simulink model "x64_32s_001"  (Simulink 7.4)
settings: Solver=ode45  StartTime=0.0  StopTime=100000  RelTol=1e-3
undefined symbols (not defined in the file): gcbh, ROACH, x64_32s_001_ct_sel_reg_user_data_out, x64_32s_001_inst_seng_id0_user_data_in, x64_32s_001_qdr_in_cnt_user_data_in, x64_32s_001_status_user_data_in, x64_32s_001_xaui_cnt0_user_data_in, x64_32s_001_xaui_error_cnt0_user_data_in, x64_32s_001_xauibuf_out_cnt_user_data_in, x64_32s_001_xauibuf_window_cnt_user_data_in, xlBlockLoadCallback
libraries: xbsIndex_r4 x7380, casper_library_misc x261, casper_library_delays x16, xps_library x6, ox_lib x1
(part 9 of 91)

== system x64_32s_001/vacc_monitor1 ==
  cnt_rst : Inport
  error0 : Inport  Port=2
  Delay25 : Subsystem  mask(pipeline){latency=1}
      mask help:
      | An explicitly laid-out delay line to avoid mapping to SRL16s
      | for use in pipelining to help achieve timing closure.
      mask init:
      | pipeline_init(gcb, 'latency', latency);
      mask Display:
      | port_label('input', 1, 'd');
      | port_label('output', 1, 'q');
      | disp(['Z^-', num2str(latency)]);
  payload_cnt1 : S-Function  LoadFcn=xlBlockLoadCallback(gcbh);  FunctionName=sysgen  Parameters=ptable_  EnableBusSupport=off  mask(Xilinx Counter Block){infoedit=Hardware notes: Free running counters are the least expensive in hardware.  A count limited counter is implemented by combining a counter with a comparator., cnt_type_sgadvanced=, cnt_type=Free Running, cnt_to=0, operation_sgadvanced=, operation=Up, start_count=0, cnt_by_val=1, arith_type_sgadvanced=, arith_type=Unsigned, n_bits=32, bin_pt=0, load_pin_sgadvanced=, load_pin=off, rst_sgadvanced=, rst=on, en_sgadvanced=, en=on, explicit_period_sgadvanced=, explicit_period=off, period=1, dbl_ovrd_sgadvanced=, dbl_ovrd=off, use_behavioral_HDL_sgadvanced=, use_behavioral_HDL=off, implementation_sgadvanced=, implementation=Fabric, xl_use_area_sgadvanced=, xl_use_area=off, xl_area=[17 32 0 32 0 0 0], use_rpm=on, xlcconfig=, has_advanced_control=0, sggui_pos=20,20,348,618, block_type=counter, block_version=10.1.3, sg_icon_stat=30,35,2,1,white,blue,0,d605779c,right,, sg_mask_display=|, sg_list_contents=, sg_blockgui_xml=}
      mask sg_mask_display=|
      | fprintf('','COMMENT: begin icon graphics');
      | patch([0 1 1 0 ],[0 0 1 1 ],[0.77 0.82 0.91]);
      | patch([0.233333 0.0666667 0.3 0.0666667 0.233333 0.5 0.566667 0.633333 0.9 0.666667 0.466667 0.3 0.5 0.3 0.466667 0.666667 0.9 0.633333 0.566667 0.5 0.233333 ],[0.171429 0.314286 0.514286 0.714286 0.857143 0.857143 0.8 0.857143 0.857143 0.657143 0.828571 0.685714 0.514286 0.342857 0.2 0.371429 0.171429 0.171429 0.228571 0.171429 0.171429 ],[0.98 0.96 0.92]);
      | plot([0 1 1 0 0 ],[0 0 1 1 0 ]);
      | fprintf('','COMMENT: end icon graphics');
      | fprintf('','COMMENT: begin icon text');
      | color('black');port_label('input',1,'rst');
      | color('black');port_label('input',2,'en');
      | color('black');port_label('output',1,'out');
      | fprintf('','COMMENT: end icon text');
      mask prompts: infoedit ""; cnt_type_sgadvanced ""; cnt_type "Counter type"; cnt_to "Count to value"; operation_sgadvanced ""; operation "Count direction"; start_count "Initial value"; cnt_by_val "Step"; arith_type_sgadvanced ""; arith_type "Output type"; n_bits "Number of bits"; bin_pt "Binary point"; load_pin_sgadvanced ""; load_pin "Provide load port"; rst_sgadvanced ""; rst "Provide synchronous reset port"; en_sgadvanced ""; en "Provide enable port"; explicit_period_sgadvanced ""; explicit_period "Sample period source"; period "Explicit period"; dbl_ovrd_sgadvanced ""; dbl_ovrd "Override with doubles"; use_behavioral_HDL_sgadvanced ""; use_behavioral_HDL "Use behavioral HDL (otherwise use core)"; implementation_sgadvanced ""; implementation "Implement using"; xl_use_area_sgadvanced ""; xl_use_area "Define FPGA area for resource estimation"; xl_area "FPGA area [slices, FFs, BRAMs, LUTs, IOBs, emb. mults, TBUFs]"; use_rpm ""; xlcconfig ""; has_advanced_control ""; sggui_pos ""; block_type ""; block_version ""; sg_icon_stat ""; sg_mask_display ""; sg_list_contents ""; sg_blockgui_xml ""
      mask help: Hardware notes: Free running counters are the least expensive in hardware. A count limited counter is implemented by combining a counter with a comparator.
      mask init: same as x64_32s_001/payload_cnt1
      settings: CopyFcn=xlBlockMoveCallback(gcbh);  DeleteFcn=xlDestroyGui(gcbh);  ModelCloseFcn=xlDestroyGui(gcbh);  PreSaveFcn=xlBlockPreSaveCallback(gcbh);  PostSaveFcn=xlBlockPostSaveCallback(gcbh);  DestroyFcn=xlDestroyGui(gcbh);  OpenFcn=xlOpenGui(gcbh, 'counter_gui.xml', @counterenablement, -1)  CloseFcn=xlDestroyGui(gcbh);  MoveFcn=xlBlockMoveCallback(gcbh);
      mask Help: eval('');xlDoc('-book','sysgen','-topic','Counter');
      mask StyleString: edit,edit,popup(Free Running|Count Limited),edit,edit,popup(Up|Down|Up/Down),edit,edit,edit,popup(Signed  (2's comp)|Unsigned),edit,edit,edit,checkbox,edit,checkbox,edit,checkbox,edit,popup(on|off),edit,edit,checkbox,edit,checkbox,edit,popup(Fabric|DSP48),edit,checkbox,edit,edit,edit,edit,edit,edit,edit,edit,edit,edit,edit
      mask Display:
      | fprintf('','COMMENT: begin icon graphics');
      | patch([0 1 1 0 ],[0 0 1 1 ],[0.77 0.82 0.91]);
      | patch([0.233333 0.0666667 0.3 0.0666667 0.233333 0.5 0.566667 0.633333 0.9 0.666667 0.466667 0.3 0.5 0.3 0.466667 0.666667 0.9 0.633333 0.566667 0.5 0.233333 ],[0.171429 0.314286 0.514286 0.714286 0.857143 0.857143 0.8 0.857143 0.857143 0.657143 0.828571 0.685714 0.514286 0.342857 0.2 0.371429 0.171429 0.171429 0.228571 0.171429 0.171429 ],[0.98 0.96 0.92]);
      | plot([0 1 1 0 0 ],[0 0 1 1 0 ]);
      | fprintf('','COMMENT: end icon graphics');
      | fprintf('','COMMENT: begin icon text');
      | color('black');port_label('input',1,'rst');
      | color('black');port_label('input',2,'en');
      | color('black');port_label('output',1,'out');
      | fprintf('','COMMENT: end icon text');
  cnt0 : Outport
  wires:
  error0/1 -> payload_cnt1/2
  Delay25/1(q) -> cnt0/1
  cnt_rst/1 -> payload_cnt1/1
  payload_cnt1/1 -> Delay25/1(d)

== system x64_32s_001/vacc_monitor2 ==
  cnt_rst : Inport
  error0 : Inport  Port=2
  Delay25 : Subsystem  mask(pipeline){latency=1}
      mask help:
      | An explicitly laid-out delay line to avoid mapping to SRL16s
      | for use in pipelining to help achieve timing closure.
      mask init:
      | pipeline_init(gcb, 'latency', latency);
      mask Display:
      | port_label('input', 1, 'd');
      | port_label('output', 1, 'q');
      | disp(['Z^-', num2str(latency)]);
  payload_cnt1 : S-Function  LoadFcn=xlBlockLoadCallback(gcbh);  FunctionName=sysgen  Parameters=ptable_  EnableBusSupport=off  mask(Xilinx Counter Block){infoedit=Hardware notes: Free running counters are the least expensive in hardware.  A count limited counter is implemented by combining a counter with a comparator., cnt_type_sgadvanced=, cnt_type=Free Running, cnt_to=0, operation_sgadvanced=, operation=Up, start_count=0, cnt_by_val=1, arith_type_sgadvanced=, arith_type=Unsigned, n_bits=32, bin_pt=0, load_pin_sgadvanced=, load_pin=off, rst_sgadvanced=, rst=on, en_sgadvanced=, en=on, explicit_period_sgadvanced=, explicit_period=off, period=1, dbl_ovrd_sgadvanced=, dbl_ovrd=off, use_behavioral_HDL_sgadvanced=, use_behavioral_HDL=off, implementation_sgadvanced=, implementation=Fabric, xl_use_area_sgadvanced=, xl_use_area=off, xl_area=[17 32 0 32 0 0 0], use_rpm=on, xlcconfig=, has_advanced_control=0, sggui_pos=20,20,348,618, block_type=counter, block_version=10.1.3, sg_icon_stat=30,35,2,1,white,blue,0,d605779c,right,, sg_mask_display=|, sg_list_contents=, sg_blockgui_xml=}
      mask sg_mask_display=|
      | fprintf('','COMMENT: begin icon graphics');
      | patch([0 1 1 0 ],[0 0 1 1 ],[0.77 0.82 0.91]);
      | patch([0.233333 0.0666667 0.3 0.0666667 0.233333 0.5 0.566667 0.633333 0.9 0.666667 0.466667 0.3 0.5 0.3 0.466667 0.666667 0.9 0.633333 0.566667 0.5 0.233333 ],[0.171429 0.314286 0.514286 0.714286 0.857143 0.857143 0.8 0.857143 0.857143 0.657143 0.828571 0.685714 0.514286 0.342857 0.2 0.371429 0.171429 0.171429 0.228571 0.171429 0.171429 ],[0.98 0.96 0.92]);
      | plot([0 1 1 0 0 ],[0 0 1 1 0 ]);
      | fprintf('','COMMENT: end icon graphics');
      | fprintf('','COMMENT: begin icon text');
      | color('black');port_label('input',1,'rst');
      | color('black');port_label('input',2,'en');
      | color('black');port_label('output',1,'out');
      | fprintf('','COMMENT: end icon text');
      mask prompts: infoedit ""; cnt_type_sgadvanced ""; cnt_type "Counter type"; cnt_to "Count to value"; operation_sgadvanced ""; operation "Count direction"; start_count "Initial value"; cnt_by_val "Step"; arith_type_sgadvanced ""; arith_type "Output type"; n_bits "Number of bits"; bin_pt "Binary point"; load_pin_sgadvanced ""; load_pin "Provide load port"; rst_sgadvanced ""; rst "Provide synchronous reset port"; en_sgadvanced ""; en "Provide enable port"; explicit_period_sgadvanced ""; explicit_period "Sample period source"; period "Explicit period"; dbl_ovrd_sgadvanced ""; dbl_ovrd "Override with doubles"; use_behavioral_HDL_sgadvanced ""; use_behavioral_HDL "Use behavioral HDL (otherwise use core)"; implementation_sgadvanced ""; implementation "Implement using"; xl_use_area_sgadvanced ""; xl_use_area "Define FPGA area for resource estimation"; xl_area "FPGA area [slices, FFs, BRAMs, LUTs, IOBs, emb. mults, TBUFs]"; use_rpm ""; xlcconfig ""; has_advanced_control ""; sggui_pos ""; block_type ""; block_version ""; sg_icon_stat ""; sg_mask_display ""; sg_list_contents ""; sg_blockgui_xml ""
      mask help: Hardware notes: Free running counters are the least expensive in hardware. A count limited counter is implemented by combining a counter with a comparator.
      mask init: same as x64_32s_001/payload_cnt1
      settings: CopyFcn=xlBlockMoveCallback(gcbh);  DeleteFcn=xlDestroyGui(gcbh);  ModelCloseFcn=xlDestroyGui(gcbh);  PreSaveFcn=xlBlockPreSaveCallback(gcbh);  PostSaveFcn=xlBlockPostSaveCallback(gcbh);  DestroyFcn=xlDestroyGui(gcbh);  OpenFcn=xlOpenGui(gcbh, 'counter_gui.xml', @counterenablement, -1)  CloseFcn=xlDestroyGui(gcbh);  MoveFcn=xlBlockMoveCallback(gcbh);
      mask Help: eval('');xlDoc('-book','sysgen','-topic','Counter');
      mask StyleString: edit,edit,popup(Free Running|Count Limited),edit,edit,popup(Up|Down|Up/Down),edit,edit,edit,popup(Signed  (2's comp)|Unsigned),edit,edit,edit,checkbox,edit,checkbox,edit,checkbox,edit,popup(on|off),edit,edit,checkbox,edit,checkbox,edit,popup(Fabric|DSP48),edit,checkbox,edit,edit,edit,edit,edit,edit,edit,edit,edit,edit,edit
      mask Display:
      | fprintf('','COMMENT: begin icon graphics');
      | patch([0 1 1 0 ],[0 0 1 1 ],[0.77 0.82 0.91]);
      | patch([0.233333 0.0666667 0.3 0.0666667 0.233333 0.5 0.566667 0.633333 0.9 0.666667 0.466667 0.3 0.5 0.3 0.466667 0.666667 0.9 0.633333 0.566667 0.5 0.233333 ],[0.171429 0.314286 0.514286 0.714286 0.857143 0.857143 0.8 0.857143 0.857143 0.657143 0.828571 0.685714 0.514286 0.342857 0.2 0.371429 0.171429 0.171429 0.228571 0.171429 0.171429 ],[0.98 0.96 0.92]);
      | plot([0 1 1 0 0 ],[0 0 1 1 0 ]);
      | fprintf('','COMMENT: end icon graphics');
      | fprintf('','COMMENT: begin icon text');
      | color('black');port_label('input',1,'rst');
      | color('black');port_label('input',2,'en');
      | color('black');port_label('output',1,'out');
      | fprintf('','COMMENT: end icon text');
  cnt0 : Outport
  wires:
  payload_cnt1/1 -> Delay25/1(d)
  cnt_rst/1 -> payload_cnt1/1
  Delay25/1(q) -> cnt0/1
  error0/1 -> payload_cnt1/2

== system x64_32s_001/vacc_sim_mux ==
  sync : Inport  OutDataType=fixdt(1, 16)  OutScaling=2^0
  vacc_din : Inport  Port=2  OutDataType=fixdt(1, 16)  OutScaling=2^0
  vld : Inport  Port=3  OutDataType=fixdt(1, 16)  OutScaling=2^0
  Counter : xbsIndex_r4/Counter  cnt_type=Free Running  cnt_to=Inf  operation=Up  start_count=0  cnt_by_val=1  arith_type=Unsigned  n_bits=3  bin_pt=0  load_pin=off  rst=on  en=off  explicit_period=on  period=1  dbl_ovrd=off  use_behavioral_HDL=off  implementation=Fabric  block_type=counter
      settings: block_version=11.4
  dout_sim : xbsIndex_r4/Simulation Multiplexer  sim_sel=1  hw_sel=2  block_type=simmux
      settings: infoedit="Distinguish input subsystems as \"simulation only\" and \"generation only\".  The input specified For Simulation will be used during Simulink simulation.  The input specified For Generation will be used during code generation.  This block will typically be used on the output of a HW Co-Simulation, Black Box, or ModelSim subsystem.<br><br>Hardware Notes: This block costs nothing."  block_version=11.4
  duplicate_16 : Subsystem
  pulse_ext : casper_library_misc/pulse_ext  SystemSampleTime=-1  pulse_len=8
  valid_sim : xbsIndex_r4/Simulation Multiplexer  sim_sel=1  hw_sel=2  block_type=simmux
      settings: infoedit="Distinguish input subsystems as \"simulation only\" and \"generation only\".  The input specified For Simulation will be used during Simulink simulation.  The input specified For Generation will be used during code generation.  This block will typically be used on the output of a HW Co-Simulation, Black Box, or ModelSim subsystem.<br><br>Hardware Notes: This block costs nothing."  block_version=11.4
  sync_out : Outport
  dout : Outport  Port=2
  vld_out : Outport  Port=3
  wires:
  vld/1 -> valid_sim/2
  valid_sim/1 -> vld_out/1
  sync/1 -> sync_out/1, Counter/1, pulse_ext/1
  dout_sim/1 -> dout/1
  vacc_din/1 -> dout_sim/2
  Counter/1 -> duplicate_16/1(In1)
  pulse_ext/1 -> valid_sim/1
  duplicate_16/1(Out1) -> dout_sim/1

== system x64_32s_001/x_window ==
  sync : Inport
  In1 : Inport  Port=2
  In2 : Inport  Port=3
  In3 : Inport  Port=4
  In4 : Inport  Port=5
  Delay : xbsIndex_r4/Delay  en=off  latency=5  dbl_ovrd=off  reg_retiming=on  block_type=delay
      settings: xl_area=[0, 0, 0, 0, 0, 0, 0]  block_version=9.2.01
  mult_0 : Subsystem  mask{coeff_width=18, input_width=4, output_width=18}
      mask prompts: coeff_width "Coefficient width?"; input_width "Input width?"; output_width "Output width?"
  mult_1 : Subsystem  mask{coeff_width=18, input_width=4, output_width=18}
      mask prompts: coeff_width "Coefficient width?"; input_width "Input width?"; output_width "Output width?"
  mult_2 : Subsystem  mask{coeff_width=18, input_width=4, output_width=18}
      mask prompts: coeff_width "Coefficient width?"; input_width "Input width?"; output_width "Output width?"
  mult_3 : Subsystem  mask{coeff_width=18, input_width=4, output_width=18}
      mask prompts: coeff_width "Coefficient width?"; input_width "Input width?"; output_width "Output width?"
  sync_out : Outport
  Out1 : Outport  Port=2
  Out2 : Outport  Port=3
  Out3 : Outport  Port=4
  Out4 : Outport  Port=5
  wires:
  In1/1 -> mult_0/1(in)
  mult_0/1(out) -> Out1/1
  In2/1 -> mult_1/1(in)
  mult_1/1(out) -> Out2/1
  In3/1 -> mult_2/1(in)
  mult_2/1(out) -> Out3/1
  In4/1 -> mult_3/1(in)
  mult_3/1(out) -> Out4/1
  sync/1 -> Delay/1
  Delay/1 -> sync_out/1

== system x64_32s_001/xaui_TVG ==
  din : Inport
  oob : Inport  Port=2
  empty : Inport  Port=3
  vld : Inport  Port=4
  ld : Inport  Port=5
  full : Inport  Port=6
  tvg_en : Inport  Port=7
  Constant1 : xbsIndex_r4/Constant  arith_type=Boolean  const=0  n_bits=36  bin_pt=0  explicit_period=on  period=1  equ=P=C  opselect=C  inp2=PCIN>>17  opr=+  inp1=P  carry=CIN  dbl_ovrd=off  block_type=constant
      settings: block_version=11.4
  Constant2 : xbsIndex_r4/Constant  arith_type=Boolean  const=0  n_bits=36  bin_pt=0  explicit_period=on  period=1  equ=P=C  opselect=C  inp2=PCIN>>17  opr=+  inp1=P  carry=CIN  dbl_ovrd=off  block_type=constant
      settings: block_version=11.4
  Constant4 : xbsIndex_r4/Constant  arith_type=Boolean  const=0  n_bits=36  bin_pt=0  explicit_period=on  period=1  equ=P=C  opselect=C  inp2=PCIN>>17  opr=+  inp1=P  carry=CIN  dbl_ovrd=off  block_type=constant
      settings: block_version=11.4
  dout-mux : xbsIndex_r4/Mux  inputs=2  en=off  latency=1  precision=Full  arith_type=Unsigned  n_bits=16  bin_pt=14  quantization=Truncate  overflow=Wrap  dbl_ovrd=off  block_type=mux
      settings: block_version=11.4
  edge_detect : casper_library_misc/edge_detect  SystemSampleTime=-1  edge=Rising  polarity=Active High  x_in=[0 0.08 0.08 0.16 0.16 0.24 0.24 0.32 0.32 0.4]  y_in="[-1 -1  1  1  1  1  1  1  1  1]"  x_out=[0.6 0.68 0.68 0.76 0.76 0.84 0.84 0.92 0.92 1]  y_out="[-1 -1  1  1 -1 -1 -1 -1 -1 -1]"
  empty_mux : xbsIndex_r4/Mux  inputs=2  en=off  latency=1  precision=Full  arith_type=Unsigned  n_bits=16  bin_pt=14  quantization=Truncate  overflow=Wrap  dbl_ovrd=off  block_type=mux
      settings: block_version=11.4
  full_mux : xbsIndex_r4/Mux  inputs=2  en=off  latency=1  precision=Full  arith_type=Unsigned  n_bits=16  bin_pt=14  quantization=Truncate  overflow=Wrap  dbl_ovrd=off  block_type=mux
      settings: block_version=11.4
  linkdown_mux : xbsIndex_r4/Mux  inputs=2  en=off  latency=1  precision=Full  arith_type=Unsigned  n_bits=16  bin_pt=14  quantization=Truncate  overflow=Wrap  dbl_ovrd=off  block_type=mux
      settings: block_version=11.4
  oob_mux : xbsIndex_r4/Mux  inputs=2  en=off  latency=1  precision=Full  arith_type=Unsigned  n_bits=16  bin_pt=14  quantization=Truncate  overflow=Wrap  dbl_ovrd=off  block_type=mux
      settings: block_version=11.4
  s_eng_TVG : Subsystem
  vld_mux : xbsIndex_r4/Mux  inputs=2  en=off  latency=1  precision=Full  arith_type=Unsigned  n_bits=16  bin_pt=14  quantization=Truncate  overflow=Wrap  dbl_ovrd=off  block_type=mux
      settings: block_version=11.4
  dout : Outport
  oob_out : Outport  Port=2
  empty_out : Outport  Port=3
  vld_out : Outport  Port=4
  ld_out : Outport  Port=5
  full_out : Outport  Port=6
  wires:
  edge_detect/1 -> s_eng_TVG/1(rst)
  s_eng_TVG/1(dout) -> dout-mux/3
  s_eng_TVG/2(vld) -> oob_mux/3
  s_eng_TVG/3(oob) -> vld_mux/3
  tvg_en/1 -> dout-mux/1, oob_mux/1, vld_mux/1, empty_mux/1, edge_detect/1, linkdown_mux/1, full_mux/1
  din/1 -> dout-mux/2
  dout-mux/1 -> dout/1
  empty/1 -> empty_mux/2
  empty_mux/1 -> empty_out/1
  linkdown_mux/1 -> ld_out/1
  full_mux/1 -> full_out/1
  ld/1 -> linkdown_mux/2
  full/1 -> full_mux/2
  Constant4/1 -> empty_mux/3
  Constant1/1 -> linkdown_mux/3
  Constant2/1 -> full_mux/3
  vld_mux/1 -> oob_out/1
  oob_mux/1 -> vld_out/1
  vld/1 -> oob_mux/2
  oob/1 -> vld_mux/2

== system x64_32s_001/xaui_buf0 ==
  din : Inport
  valid : Inport  Port=2
  hdr_valid : Inport  Port=3
  eof_in : Inport  Port=4
  hdr_in : Inport  Port=5
  Constant8 : xbsIndex_r4/Constant  arith_type=Boolean  const=0  n_bits=1  bin_pt=0  explicit_period=on  period=1  equ=P=C  opselect=C  inp2=PCIN>>17  opr=+  inp1=P  carry=CIN  dbl_ovrd=off  block_type=constant
  Delay39 : Subsystem  AncestorBlock=casper_library/Delays/pipeline  mask(pipeline){latency=1}
      mask help:
      | An explicitly laid-out delay line to avoid mapping to SRL16s
      | for use in pipelining to help achieve timing closure.
      mask init:
      | pipeline_init(gcb, 'latency', latency);
      mask Display:
      | port_label('input', 1, 'd');
      | port_label('output', 1, 'q');
      | disp(['Z^-', num2str(latency)]);
  Delay40 : Subsystem  AncestorBlock=casper_library/Delays/pipeline  mask(pipeline){latency=1}
      mask help:
      | An explicitly laid-out delay line to avoid mapping to SRL16s
      | for use in pipelining to help achieve timing closure.
      mask init:
      | pipeline_init(gcb, 'latency', latency);
      mask Display:
      | port_label('input', 1, 'd');
      | port_label('output', 1, 'q');
      | disp(['Z^-', num2str(latency)]);
  Inverter : xbsIndex_r4/Inverter  en=off  latency=0  dbl_ovrd=off  block_type=inv
      settings: block_version=11.4
  Logical1 : xbsIndex_r4/Logical  logical_function=AND  inputs=2  en=off  latency=1  precision=Full  arith_type=Unsigned  n_bits=8  bin_pt=2  align_bp=on  dbl_ovrd=off  block_type=logical
      settings: xl_area=[0, 0, 0, 0, 0, 0, 0]
  Slice1 : xbsIndex_r4/Slice  nbits=48  boolean_output=off  mode=Lower Bit Location + Width  bit1=0  base1=MSB of Input  bit0=16  base0=LSB of Input  dbl_ovrd=off  block_type=slice
      settings: infoedit="Extracts a given range of bits from each input sample and presents it at the output.  The output type is ordinarily unsigned with binary point at zero, but can be Boolean when the slice is one bit wide.<P><P>Hardware notes: In hardware this block costs nothing."  block_version=10.1.3
  Slice3 : xbsIndex_r4/Slice  nbits=16  boolean_output=off  mode=Lower Bit Location + Width  bit1=0  base1=MSB of Input  bit0=0  base0=LSB of Input  dbl_ovrd=off  block_type=slice
      settings: infoedit="Extracts a given range of bits from each input sample and presents it at the output.  The output type is ordinarily unsigned with binary point at zero, but can be Boolean when the slice is one bit wide.<P><P>Hardware notes: In hardware this block costs nothing."  block_version=10.1.3
  pkt_hdr : xbsIndex_r4/Register  init=0  rst=off  en=on  dbl_ovrd=off  block_type=register
      settings: xl_area=[0, 0, 0, 0, 0, 0, 0]
  dout : Outport
  we : Outport  Port=2
  eof_out : Outport  Port=3
  mcnt : Outport  Port=4
  ant : Outport  Port=5
  flag : Outport  Port=6
  wires:
  hdr_in/1 -> pkt_hdr/1
  Constant8/1 -> flag/1
  Slice3/1 -> ant/1
  Slice1/1 -> mcnt/1
  Delay39/1(q) -> eof_out/1
  eof_in/1 -> Delay39/1(d)
  Logical1/1 -> we/1
  valid/1 -> Logical1/1
  Delay40/1(q) -> dout/1
  din/1 -> Delay40/1(d)
  Inverter/1 -> Logical1/2
  hdr_valid/1 -> pkt_hdr/2, Inverter/1
  pkt_hdr/1 -> Slice3/1, Slice1/1

== system x64_32s_001/xaui_cnt0 ==
  reg_out : Inport  OutDataType=sfix(16)  OutScaling=2^0
  convert : xbsIndex_r4/Convert  arith_type=Unsigned  n_bits=32  bin_pt=0  quantization=Truncate  overflow=Wrap  latency=0  dbl_ovrd=off  pipeline=off  block_type=convert
      settings: block_version=11.4
  x64_32s_001_xaui_cnt0_user_data_in : xbsIndex_r4/Gateway Out  hdl_port=on  timing_constraint=None  locs_specified=off  LOCs={}  block_type=gatewayout
      settings: infoedit="Gateway out block.  Converts Xilinx fixed point inputs into ouputs of type Simulink integer, double, or fixed point.<br><br>Hardware notes:  In hardware these blocks become top level output ports or are discarded, depending on how they are configured."  block_version=11.4
  sim_out : Outport  OutDataType=sfix(16)  OutScaling=2^0
  wires:
  reg_out/1 -> convert/1
  convert/1 -> x64_32s_001_xaui_cnt0_user_data_in/1
  x64_32s_001_xaui_cnt0_user_data_in/1 -> sim_out/1

== system x64_32s_001/xaui_error_cnt0 ==
  reg_out : Inport  OutDataType=sfix(16)  OutScaling=2^0
  convert : xbsIndex_r4/Convert  arith_type=Unsigned  n_bits=32  bin_pt=0  quantization=Truncate  overflow=Wrap  latency=0  dbl_ovrd=off  pipeline=off  block_type=convert
      settings: block_version=11.4
  x64_32s_001_xaui_error_cnt0_user_data_in : xbsIndex_r4/Gateway Out  hdl_port=on  timing_constraint=None  locs_specified=off  LOCs={}  block_type=gatewayout
      settings: infoedit="Gateway out block.  Converts Xilinx fixed point inputs into ouputs of type Simulink integer, double, or fixed point.<br><br>Hardware notes:  In hardware these blocks become top level output ports or are discarded, depending on how they are configured."  block_version=11.4
  sim_out : Outport  OutDataType=sfix(16)  OutScaling=2^0
  wires:
  reg_out/1 -> convert/1
  convert/1 -> x64_32s_001_xaui_error_cnt0_user_data_in/1
  x64_32s_001_xaui_error_cnt0_user_data_in/1 -> sim_out/1

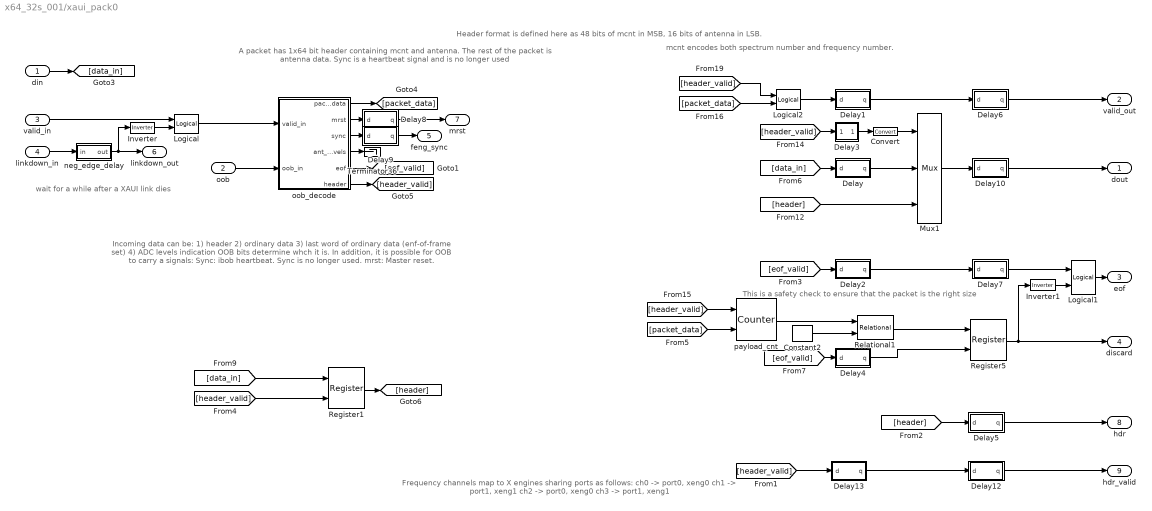
== system x64_32s_001/xaui_pack0 ==
  note: Frequency channels map to
      | X engines sharing ports as follows:
      |
      | ch0 -> port0, xeng0
      | ch1 -> port1, xeng1
      | ch2 -> port0, xeng0
      | ch3 -> port1, xeng1
  note: Header format is defined here
      | as 48 bits of mcnt in MSB, 16 bits of
      | antenna in LSB.
  note: Incoming data can be:
      | 1) header
      | 2) ordinary data
      | 3) last word of ordinary data (enf-of-frame set)
      | 4) ADC levels indication
      |  OOB bits determine whch it is.
      | In addition, it is possible for OOB to carry a signals:
      | Sync: ibob heartbeat. Sync is no longer used.
      | mrst: Master reset.
  note: wait for a while after a XAUI link dies
  note: This is a safety check to
      | ensure that the packet is the right size
  note: A packet has 1x64 bit header
      | containing mcnt and antenna.
      | The rest of the packet is antenna data.
      |
      | Sync is a heartbeat signal and is no longer used
  note: mcnt encodes both spectrum number
      | and frequency number.
  din : Inport  OutDataType=sfix(16)  OutScaling=2^0
  oob : Inport  Port=2  OutDataType=sfix(16)  OutScaling=2^0
  valid_in : Inport  Port=3  OutDataType=sfix(16)  OutScaling=2^0
  linkdown_in : Inport  Port=4  OutDataType=sfix(16)  OutScaling=2^0
  Constant2 : xbsIndex_r4/Constant  arith_type=Unsigned  const=packet_len-1  n_bits=packet_bits+1  bin_pt=0  explicit_period=on  period=1  equ=P=C  opselect=C  inp2=PCIN>>17  opr=+  inp1=P  carry=CIN  dbl_ovrd=off  block_type=constant
  Convert : xbsIndex_r4/Convert  arith_type=Unsigned  n_bits=1  bin_pt=0  quantization=Truncate  overflow=Wrap  latency=0  dbl_ovrd=off  pipeline=off  block_type=convert
      settings: xl_area=[0, 0, 0, 0, 0, 0, 0]  block_version=10.1.3
  Delay : Subsystem  AncestorBlock=casper_library/Delays/pipeline  mask(pipeline){latency=1}
      mask help:
      | An explicitly laid-out delay line to avoid mapping to SRL16s
      | for use in pipelining to help achieve timing closure.
      mask init:
      | pipeline_init(gcb, 'latency', latency);
      mask Display:
      | port_label('input', 1, 'd');
      | port_label('output', 1, 'q');
      | disp(['Z^-', num2str(latency)]);
  Delay1 : Subsystem  AncestorBlock=casper_library/Delays/pipeline  mask(pipeline){latency=1}
      mask help:
      | An explicitly laid-out delay line to avoid mapping to SRL16s
      | for use in pipelining to help achieve timing closure.
      mask init:
      | pipeline_init(gcb, 'latency', latency);
      mask Display:
      | port_label('input', 1, 'd');
      | port_label('output', 1, 'q');
      | disp(['Z^-', num2str(latency)]);
  Delay10 : Subsystem  AncestorBlock=casper_library/Delays/pipeline  mask(pipeline){latency=1}
      mask help:
      | An explicitly laid-out delay line to avoid mapping to SRL16s
      | for use in pipelining to help achieve timing closure.
      mask init:
      | pipeline_init(gcb, 'latency', latency);
      mask Display:
      | port_label('input', 1, 'd');
      | port_label('output', 1, 'q');
      | disp(['Z^-', num2str(latency)]);
  Delay12 : Subsystem  AncestorBlock=casper_library/Delays/pipeline  mask(pipeline){latency=1}
      mask help:
      | An explicitly laid-out delay line to avoid mapping to SRL16s
      | for use in pipelining to help achieve timing closure.
      mask init:
      | pipeline_init(gcb, 'latency', latency);
      mask Display:
      | port_label('input', 1, 'd');
      | port_label('output', 1, 'q');
      | disp(['Z^-', num2str(latency)]);
  Delay13 : Subsystem  AncestorBlock=casper_library/Delays/pipeline  mask(pipeline){latency=1}
      mask help:
      | An explicitly laid-out delay line to avoid mapping to SRL16s
      | for use in pipelining to help achieve timing closure.
      mask init:
      | pipeline_init(gcb, 'latency', latency);
      mask Display:
      | port_label('input', 1, 'd');
      | port_label('output', 1, 'q');
      | disp(['Z^-', num2str(latency)]);
  Delay2 : Subsystem  AncestorBlock=casper_library/Delays/pipeline  mask(pipeline){latency=1}
      mask help:
      | An explicitly laid-out delay line to avoid mapping to SRL16s
      | for use in pipelining to help achieve timing closure.
      mask init:
      | pipeline_init(gcb, 'latency', latency);
      mask Display:
      | port_label('input', 1, 'd');
      | port_label('output', 1, 'q');
      | disp(['Z^-', num2str(latency)]);
  Delay3 : Subsystem  AncestorBlock=casper_library/Delays/pipeline  mask(pipeline){latency=1}
      mask help:
      | An explicitly laid-out delay line to avoid mapping to SRL16s
      | for use in pipelining to help achieve timing closure.
      mask init:
      | pipeline_init(gcb, 'latency', latency);
      mask Display:
      | port_label('input', 1, 'd');
      | port_label('output', 1, 'q');
      | disp(['Z^-', num2str(latency)]);
  Delay4 : Subsystem  AncestorBlock=casper_library/Delays/pipeline  mask(pipeline){latency=1}
      mask help:
      | An explicitly laid-out delay line to avoid mapping to SRL16s
      | for use in pipelining to help achieve timing closure.
      mask init:
      | pipeline_init(gcb, 'latency', latency);
      mask Display:
      | port_label('input', 1, 'd');
      | port_label('output', 1, 'q');
      | disp(['Z^-', num2str(latency)]);
  Delay5 : Subsystem  AncestorBlock=casper_library/Delays/pipeline  mask(pipeline){latency=1}
      mask help:
      | An explicitly laid-out delay line to avoid mapping to SRL16s
      | for use in pipelining to help achieve timing closure.
      mask init:
      | pipeline_init(gcb, 'latency', latency);
      mask Display:
      | port_label('input', 1, 'd');
      | port_label('output', 1, 'q');
      | disp(['Z^-', num2str(latency)]);
  Delay6 : Subsystem  AncestorBlock=casper_library/Delays/pipeline  mask(pipeline){latency=1}
      mask help:
      | An explicitly laid-out delay line to avoid mapping to SRL16s
      | for use in pipelining to help achieve timing closure.
      mask init:
      | pipeline_init(gcb, 'latency', latency);
      mask Display:
      | port_label('input', 1, 'd');
      | port_label('output', 1, 'q');
      | disp(['Z^-', num2str(latency)]);
  Delay7 : Subsystem  AncestorBlock=casper_library/Delays/pipeline  mask(pipeline){latency=1}
      mask help:
      | An explicitly laid-out delay line to avoid mapping to SRL16s
      | for use in pipelining to help achieve timing closure.
      mask init:
      | pipeline_init(gcb, 'latency', latency);
      mask Display:
      | port_label('input', 1, 'd');
      | port_label('output', 1, 'q');
      | disp(['Z^-', num2str(latency)]);
  Delay8 : Subsystem  AncestorBlock=casper_library/Delays/pipeline  mask(pipeline){latency=1}
      mask help:
      | An explicitly laid-out delay line to avoid mapping to SRL16s
      | for use in pipelining to help achieve timing closure.
      mask init:
      | pipeline_init(gcb, 'latency', latency);
      mask Display:
      | port_label('input', 1, 'd');
      | port_label('output', 1, 'q');
      | disp(['Z^-', num2str(latency)]);
  Delay9 : Subsystem  AncestorBlock=casper_library/Delays/pipeline  mask(pipeline){latency=2}
      mask help:
      | An explicitly laid-out delay line to avoid mapping to SRL16s
      | for use in pipelining to help achieve timing closure.
      mask init:
      | pipeline_init(gcb, 'latency', latency);
      mask Display:
      | port_label('input', 1, 'd');
      | port_label('output', 1, 'q');
      | disp(['Z^-', num2str(latency)]);
  Inverter : xbsIndex_r4/Inverter  en=off  latency=0  dbl_ovrd=off  block_type=inv
      settings: xl_area=[0, 0, 0, 0, 0, 0, 0]
  Inverter1 : xbsIndex_r4/Inverter  en=off  latency=0  dbl_ovrd=off  block_type=inv
      settings: xl_area=[0, 0, 0, 0, 0, 0, 0]
  Logical : xbsIndex_r4/Logical  logical_function=AND  inputs=2  en=off  latency=0  precision=Full  arith_type=Unsigned  n_bits=8  bin_pt=2  align_bp=on  dbl_ovrd=off  block_type=logical
      settings: xl_area=[0, 0, 0, 0, 0, 0, 0]
  Logical1 : xbsIndex_r4/Logical  logical_function=AND  inputs=2  en=off  latency=0  precision=Full  arith_type=Unsigned  n_bits=8  bin_pt=2  align_bp=on  dbl_ovrd=off  block_type=logical
      settings: xl_area=[0, 0, 0, 0, 0, 0, 0]
  Logical2 : xbsIndex_r4/Logical  logical_function=OR  inputs=2  en=off  latency=0  precision=Full  arith_type=Unsigned  n_bits=8  bin_pt=2  align_bp=on  dbl_ovrd=off  block_type=logical
      settings: xl_area=[0, 0, 0, 0, 0, 0, 0]
  Mux1 : xbsIndex_r4/Mux  inputs=2  en=off  latency=0  precision=Full  arith_type="Signed  (2's comp)"  n_bits=8  bin_pt=2  quantization=Truncate  overflow=Wrap  dbl_ovrd=off  block_type=mux
      settings: xl_area=[0, 0, 0, 0, 0, 0, 0]
  Register1 : xbsIndex_r4/Register  init=0  rst=off  en=on  dbl_ovrd=off  block_type=register
      settings: xl_area=[0, 0, 0, 0, 0, 0, 0]
  Register5 : xbsIndex_r4/Register  init=0  rst=off  en=on  dbl_ovrd=off  block_type=register
      settings: xl_area=[0, 0, 0, 0, 0, 0, 0]
  Relational1 : xbsIndex_r4/Relational  mode=a!=b  en=off  latency=0  dbl_ovrd=off  block_type=relational
      settings: xl_area=[0, 0, 0, 0, 0, 0, 0]  block_version=10.1.3
  Terminator36 : Terminator
  neg_edge_delay : Subsystem  AncestorBlock=casper_library/Misc/pulse_ext  mask(pulse_ext){pulse_len=5}
      mask prompts: pulse_len "Length of Pulse"
      mask help:
      | Extends a boolean signal to be high for the specified number of clocks after
      | the last high input.
      mask init:
      | bits = ceil(log2(pulse_len+1));
      mask Help: eval('xlWeb([getenv(''MLIB_ROOT''), ''\casper_library\doc\pulse_ext_usage.html''])')
  oob_decode : Subsystem
  payload_cnt : xbsIndex_r4/Counter  cnt_type=Free Running  cnt_to=packet_len - 1  operation=Up  start_count=0  cnt_by_val=1  arith_type=Unsigned  n_bits=packet_bits+1  bin_pt=0  load_pin=off  rst=on  en=on  explicit_period=off  period=1  dbl_ovrd=off  use_behavioral_HDL=off  implementation=Fabric  use_rpm=on  block_type=counter
      settings: xl_area=[0, 0, 0, 0, 0, 0, 0]
  dout : Outport  OutDataType=sfix(16)  OutScaling=2^0
  valid_out : Outport  Port=2  OutDataType=sfix(16)  OutScaling=2^0
  eof : Outport  Port=3  OutDataType=sfix(16)  OutScaling=2^0
  discard : Outport  Port=4  OutDataType=sfix(16)  OutScaling=2^0
  feng_sync : Outport  Port=5  OutDataType=sfix(16)  OutScaling=2^0
  linkdown_out : Outport  Port=6  OutDataType=sfix(16)  OutScaling=2^0
  mrst : Outport  Port=7  OutDataType=sfix(16)  OutScaling=2^0
  hdr : Outport  Port=8  OutDataType=sfix(16)  OutScaling=2^0
  hdr_valid : Outport  Port=9  OutDataType=sfix(16)  OutScaling=2^0
  wires:
  [eof_valid] -> Delay4/1(d)
  [eof_valid] -> Delay2/1(d)
  oob_decode/5(eof) -> [eof_valid]
  [header] -> Delay5/1(d)
  [header_valid] -> Delay13/1(d)
  Delay13/1(q) -> Delay12/1(d)
  Delay12/1(q) -> hdr_valid/1
  Delay5/1(q) -> hdr/1
  Delay10/1(q) -> dout/1
  Delay7/1(q) -> Logical1/1
  Logical1/1 -> eof/1
  Inverter1/1 -> Logical1/2
  Delay9/1(q) -> feng_sync/1
  Delay8/1(q) -> mrst/1
  Delay6/1(q) -> valid_out/1
  Delay4/1(q) -> Register5/2
  [header_valid] -> Logical2/1
  [packet_data] -> Logical2/2
  [data_in] -> Delay/1(d)
  Delay3/1(q) -> Convert/1
  Convert/1 -> Mux1/1
  Delay2/1(q) -> Delay7/1(d)
  Delay1/1(q) -> Delay6/1(d)
  Logical2/1 -> Delay1/1(d)
  [header] -> Mux1/3
  Register1/1 -> [header]
  [data_in] -> Register1/1
  [header_valid] -> Register1/2
  [header_valid] -> Delay3/1(d)
  Delay/1(q) -> Mux1/2
  oob_decode/6(header) -> [header_valid]
  Register5/1 -> Inverter1/1, discard/1
  Relational1/1 -> Register5/1
  oob_decode/1(packet_data) -> [packet_data]
  oob_decode/3(sync) -> Delay9/1(d)
  oob_decode/4(ant_levels) -> Terminator36/1
  oob_decode/2(mrst) -> Delay8/1(d)
  [header_valid] -> payload_cnt/1
  din/1 -> [data_in]
  oob/1 -> oob_decode/2(oob_in)
  linkdown_in/1 -> neg_edge_delay/1(in)
  Logical/1 -> oob_decode/1(valid_in)
  valid_in/1 -> Logical/1
  neg_edge_delay/1(out) -> Inverter/1, linkdown_out/1
  Inverter/1 -> Logical/2
  [packet_data] -> payload_cnt/2
  Constant2/1 -> Relational1/2
  payload_cnt/1 -> Relational1/1
  Mux1/1 -> Delay10/1(d)

== system x64_32s_001/xaui_sim_mux2 ==
  rx_data : Inport  OutDataType=fixdt(1, 16)  OutScaling=2^0
  rx_oob : Inport  Port=2  OutDataType=fixdt(1, 16)  OutScaling=2^0
  rx_empty : Inport  Port=3  OutDataType=fixdt(1, 16)  OutScaling=2^0
  rx_valid : Inport  Port=4  OutDataType=fixdt(1, 16)  OutScaling=2^0
  rx_linkdown : Inport  Port=5  OutDataType=fixdt(1, 16)  OutScaling=2^0
  tx_full : Inport  Port=6  OutDataType=fixdt(1, 16)  OutScaling=2^0
  rx_almost_full : Inport  Port=7  OutDataType=fixdt(1, 16)  OutScaling=2^0
  Concat : xbsIndex_r4/Concat  num_inputs=3  dbl_ovrd=off  block_type=concat
  Constant1 : xbsIndex_r4/Constant  arith_type=Unsigned  const=0  n_bits=6  bin_pt=0  explicit_period=on  period=1  equ=P=C  opselect=C  inp2=PCIN>>17  opr=+  inp1=P  carry=CIN  dbl_ovrd=off  block_type=constant
      settings: block_version=11.4
  Constant24 : xbsIndex_r4/Constant  arith_type=Unsigned  const=0  n_bits=2  bin_pt=0  explicit_period=on  period=1  equ=P=C  opselect=C  inp2=PCIN>>17  opr=+  inp1=P  carry=CIN  dbl_ovrd=off  block_type=constant
      settings: block_version=11.4
  Convert : xbsIndex_r4/Convert  arith_type=Unsigned  n_bits=64  bin_pt=0  quantization=Truncate  overflow=Wrap  latency=0  dbl_ovrd=off  pipeline=off  block_type=convert
      settings: block_version=11.4
  Counter : xbsIndex_r4/Counter  cnt_type=Free Running  cnt_to=Inf  operation=Up  start_count=start_val  cnt_by_val=1  arith_type=Unsigned  n_bits=8  bin_pt=0  load_pin=off  rst=on  en=on  explicit_period=on  period=1  dbl_ovrd=off  use_behavioral_HDL=off  implementation=Fabric  block_type=counter
      settings: block_version=11.4
  Counter1 : xbsIndex_r4/Counter  cnt_type=Free Running  cnt_to=Inf  operation=Up  start_count=0  cnt_by_val=1  arith_type=Unsigned  n_bits=2  bin_pt=0  load_pin=off  rst=on  en=off  explicit_period=on  period=1  dbl_ovrd=off  use_behavioral_HDL=off  implementation=Fabric  block_type=counter
      settings: block_version=11.4
  Delay : xbsIndex_r4/Delay  en=off  latency=xaui_lat  dbl_ovrd=off  reg_retiming=off  block_type=delay
      settings: block_version=11.4
  Delay1 : xbsIndex_r4/Delay  en=off  latency=xaui_lat  dbl_ovrd=off  reg_retiming=off  block_type=delay
      settings: block_version=11.4
  Delay2 : xbsIndex_r4/Delay  en=off  latency=xaui_lat  dbl_ovrd=off  reg_retiming=off  block_type=delay
      settings: block_version=11.4
  Delay3 : xbsIndex_r4/Delay  en=off  latency=1  dbl_ovrd=off  reg_retiming=off  block_type=delay
      settings: block_version=11.4
  Relational2 : xbsIndex_r4/Relational  mode=a=b  en=off  latency=0  dbl_ovrd=off  block_type=relational
  dout_sim : xbsIndex_r4/Simulation Multiplexer  sim_sel=1  hw_sel=2  block_type=simmux
      settings: infoedit="Distinguish input subsystems as \"simulation only\" and \"generation only\".  The input specified For Simulation will be used during Simulink simulation.  The input specified For Generation will be used during code generation.  This block will typically be used on the output of a HW Co-Simulation, Black Box, or ModelSim subsystem.<br><br>Hardware Notes: This block costs nothing."  block_version=11.4
  oob_sim : xbsIndex_r4/Simulation Multiplexer  sim_sel=1  hw_sel=2  block_type=simmux
      settings: infoedit="Distinguish input subsystems as \"simulation only\" and \"generation only\".  The input specified For Simulation will be used during Simulink simulation.  The input specified For Generation will be used during code generation.  This block will typically be used on the output of a HW Co-Simulation, Black Box, or ModelSim subsystem.<br><br>Hardware Notes: This block costs nothing."  block_version=11.4
  repeat_x3 : Subsystem
  sim_trig : ox_lib/sim_trig  SystemSampleTime=-1  delay=1
  valid_sim : xbsIndex_r4/Simulation Multiplexer  sim_sel=1  hw_sel=2  block_type=simmux
      settings: infoedit="Distinguish input subsystems as \"simulation only\" and \"generation only\".  The input specified For Simulation will be used during Simulink simulation.  The input specified For Generation will be used during code generation.  This block will typically be used on the output of a HW Co-Simulation, Black Box, or ModelSim subsystem.<br><br>Hardware Notes: This block costs nothing."  block_version=11.4
  dout : Outport
  oob : Outport  Port=2
  empty : Outport  Port=3
  valid : Outport  Port=4
  linkdown : Outport  Port=5
  full : Outport  Port=6
  af : Outport  Port=7
  wires:
  Delay2/1 -> valid_sim/1
  Delay1/1 -> oob_sim/1
  Delay/1 -> dout_sim/1
  Concat/1 -> Delay1/1
  rx_valid/1 -> valid_sim/2
  Relational2/1 -> Delay2/1, Counter/2
  Constant24/1 -> Relational2/2
  Counter1/1 -> Relational2/1
  sim_trig/1 -> Counter1/1, Delay3/1, Counter/1
  valid_sim/1 -> valid/1
  dout_sim/1 -> dout/1
  rx_almost_full/1 -> af/1
  rx_linkdown/1 -> linkdown/1
  tx_full/1 -> full/1
  rx_data/1 -> dout_sim/2
  rx_empty/1 -> empty/1
  oob_sim/1 -> oob/1
  rx_oob/1 -> oob_sim/2
  Counter/1 -> Convert/1
  Convert/1 -> Delay/1
  Delay3/1 -> Concat/1, repeat_x3/1(In1)
  Constant1/1 -> Concat/3
  ? -> ?
  repeat_x3/1(Out1) -> Concat/2

== system x64_32s_001/xauibuf_out_cnt ==
  reg_out : Inport  OutDataType=sfix(16)  OutScaling=2^0
  convert : xbsIndex_r4/Convert  arith_type=Unsigned  n_bits=32  bin_pt=0  quantization=Truncate  overflow=Wrap  latency=0  dbl_ovrd=off  pipeline=off  block_type=convert
      settings: block_version=11.4
  x64_32s_001_xauibuf_out_cnt_user_data_in : xbsIndex_r4/Gateway Out  hdl_port=on  timing_constraint=None  locs_specified=off  LOCs={}  block_type=gatewayout
      settings: infoedit="Gateway out block.  Converts Xilinx fixed point inputs into ouputs of type Simulink integer, double, or fixed point.<br><br>Hardware notes:  In hardware these blocks become top level output ports or are discarded, depending on how they are configured."  block_version=11.4
  sim_out : Outport  OutDataType=sfix(16)  OutScaling=2^0
  wires:
  reg_out/1 -> convert/1
  convert/1 -> x64_32s_001_xauibuf_out_cnt_user_data_in/1
  x64_32s_001_xauibuf_out_cnt_user_data_in/1 -> sim_out/1

== system x64_32s_001/xauibuf_window_cnt ==
  reg_out : Inport  OutDataType=sfix(16)  OutScaling=2^0
  convert : xbsIndex_r4/Convert  arith_type=Unsigned  n_bits=32  bin_pt=0  quantization=Truncate  overflow=Wrap  latency=0  dbl_ovrd=off  pipeline=off  block_type=convert
      settings: block_version=11.4
  x64_32s_001_xauibuf_window_cnt_user_data_in : xbsIndex_r4/Gateway Out  hdl_port=on  timing_constraint=None  locs_specified=off  LOCs={}  block_type=gatewayout
      settings: infoedit="Gateway out block.  Converts Xilinx fixed point inputs into ouputs of type Simulink integer, double, or fixed point.<br><br>Hardware notes:  In hardware these blocks become top level output ports or are discarded, depending on how they are configured."  block_version=11.4
  sim_out : Outport  OutDataType=sfix(16)  OutScaling=2^0
  wires:
  reg_out/1 -> convert/1
  convert/1 -> x64_32s_001_xauibuf_window_cnt_user_data_in/1
  x64_32s_001_xauibuf_window_cnt_user_data_in/1 -> sim_out/1

== system x64_32s_001/y_window ==
  sync : Inport
  In1 : Inport  Port=2
  In2 : Inport  Port=3
  In3 : Inport  Port=4
  In4 : Inport  Port=5
  In5 : Inport  Port=6
  In6 : Inport  Port=7
  In7 : Inport  Port=8
  In8 : Inport  Port=9
  Delay : xbsIndex_r4/Delay  en=off  latency=5  dbl_ovrd=off  reg_retiming=on  block_type=delay
      settings: xl_area=[0, 0, 0, 0, 0, 0, 0]  block_version=9.2.01
  mult_0 : Subsystem  mask{coeff_width=18, input_width=4, output_width=18}
      mask prompts: coeff_width "Coefficient width?"; input_width "Input width?"; output_width "Output width?"
  mult_1 : Subsystem  mask{coeff_width=18, input_width=4, output_width=18}
      mask prompts: coeff_width "Coefficient width?"; input_width "Input width?"; output_width "Output width?"
  mult_2 : Subsystem  mask{coeff_width=18, input_width=4, output_width=18}
      mask prompts: coeff_width "Coefficient width?"; input_width "Input width?"; output_width "Output width?"
  mult_3 : Subsystem  mask{coeff_width=18, input_width=4, output_width=18}
      mask prompts: coeff_width "Coefficient width?"; input_width "Input width?"; output_width "Output width?"
  mult_4 : Subsystem  mask{coeff_width=18, input_width=4, output_width=18}
      mask prompts: coeff_width "Coefficient width?"; input_width "Input width?"; output_width "Output width?"
  mult_5 : Subsystem  mask{coeff_width=18, input_width=4, output_width=18}
      mask prompts: coeff_width "Coefficient width?"; input_width "Input width?"; output_width "Output width?"
  mult_6 : Subsystem  mask{coeff_width=18, input_width=4, output_width=18}
      mask prompts: coeff_width "Coefficient width?"; input_width "Input width?"; output_width "Output width?"
  mult_7 : Subsystem  mask{coeff_width=18, input_width=4, output_width=18}
      mask prompts: coeff_width "Coefficient width?"; input_width "Input width?"; output_width "Output width?"
  sync_out : Outport
  Out1 : Outport  Port=2
  Out2 : Outport  Port=3
  Out3 : Outport  Port=4
  Out4 : Outport  Port=5
  Out5 : Outport  Port=6
  Out6 : Outport  Port=7
  Out7 : Outport  Port=8
  Out8 : Outport  Port=9
  wires:
  In1/1 -> mult_0/1(in)
  mult_0/1(out) -> Out1/1
  In2/1 -> mult_1/1(in)
  mult_1/1(out) -> Out2/1
  In3/1 -> mult_2/1(in)
  mult_2/1(out) -> Out3/1
  In4/1 -> mult_3/1(in)
  mult_3/1(out) -> Out4/1
  sync/1 -> Delay/1
  Delay/1 -> sync_out/1
  In5/1 -> mult_4/1(in)
  mult_4/1(out) -> Out5/1
  In6/1 -> mult_5/1(in)
  mult_5/1(out) -> Out6/1
  In7/1 -> mult_6/1(in)
  mult_6/1(out) -> Out7/1
  In8/1 -> mult_7/1(in)
  mult_7/1(out) -> Out8/1

== system x64_32s_001/zero_mask ==
  In1 : Inport
  In2 : Inport  Port=2
  In3 : Inport  Port=3
  In4 : Inport  Port=4
  en_mask : Inport  Port=5
  Constant{1..2,5,10} (4 alike) : xbsIndex_r4/Constant  arith_type=Unsigned  const=0  n_bits=36  bin_pt=0  explicit_period=on  period=1  equ=P=C  opselect=C  inp2=PCIN>>17  opr=+  inp1=P  carry=CIN  dbl_ovrd=off  block_type=constant
      settings: block_version=11.4
  Mux{,1..3} (4 alike) : xbsIndex_r4/Mux  inputs=2  en=off  latency=0  precision=Full  arith_type=Unsigned  n_bits=16  bin_pt=14  quantization=Truncate  overflow=Wrap  dbl_ovrd=off  block_type=mux
      settings: block_version=11.4
  uncram : Subsystem  AncestorBlock=gavrt_library/uncram  mask(uncram){num_slice=4, slice_width=1, bin_pt=0, arith_type=0}
      mask prompts: num_slice "Number of slices"; slice_width "Slice Width"; bin_pt "Output Binary Point"; arith_type "Output Arithmetic Type (0=Unsigned, 1=Signed)"
      mask help: Takes a concatenated input and slices it up into even pieces and reinterprets them as signed fixed point numbers with a given binary point.
      mask init:
      | uncram_init(gcb, ...
      |     'num_slice', num_slice, ...
      |     'slice_width', slice_width, ...
      |     'bin_pt', bin_pt, ...
      |     'arith_type', arith_type);
      settings: AttributesFormatString=UFix_1_0
  Out1 : Outport
  Out2 : Outport  Port=2
  Out3 : Outport  Port=3
  Out4 : Outport  Port=4
  wires:
  Constant5/1 -> Mux/3
  Constant10/1 -> Mux1/3
  Mux/1 -> Out1/1
  Mux1/1 -> Out2/1
  Constant2/1 -> Mux2/3
  Constant1/1 -> Mux3/3
  Mux2/1 -> Out3/1
  Mux3/1 -> Out4/1
  In1/1 -> Mux/2
  In4/1 -> Mux3/2
  In2/1 -> Mux1/2
  In3/1 -> Mux2/2
  en_mask/1 -> uncram/1(In)
  uncram/4(Out4) -> Mux/1
  uncram/3(Out3) -> Mux1/1
  uncram/2(Out2) -> Mux2/1
  uncram/1(Out1) -> Mux3/1

== system x64_32s_001/zero_mask1 ==
  In1 : Inport
  In2 : Inport  Port=2
  In3 : Inport  Port=3
  In4 : Inport  Port=4
  In5 : Inport  Port=5
  In6 : Inport  Port=6
  In7 : Inport  Port=7
  In8 : Inport  Port=8
  en_mask : Inport  Port=9
  Constant{1..7,10} (8 alike) : xbsIndex_r4/Constant  arith_type=Unsigned  const=0  n_bits=36  bin_pt=0  explicit_period=on  period=1  equ=P=C  opselect=C  inp2=PCIN>>17  opr=+  inp1=P  carry=CIN  dbl_ovrd=off  block_type=constant
      settings: block_version=11.4
  Mux{,1..7} (8 alike) : xbsIndex_r4/Mux  inputs=2  en=off  latency=0  precision=Full  arith_type=Unsigned  n_bits=16  bin_pt=14  quantization=Truncate  overflow=Wrap  dbl_ovrd=off  block_type=mux
      settings: block_version=11.4
  uncram : Subsystem  AncestorBlock=gavrt_library/uncram  mask(uncram){num_slice=8, slice_width=1, bin_pt=0, arith_type=0}
      mask prompts: num_slice "Number of slices"; slice_width "Slice Width"; bin_pt "Output Binary Point"; arith_type "Output Arithmetic Type (0=Unsigned, 1=Signed)"
      mask help: Takes a concatenated input and slices it up into even pieces and reinterprets them as signed fixed point numbers with a given binary point.
      mask init:
      | uncram_init(gcb, ...
      |     'num_slice', num_slice, ...
      |     'slice_width', slice_width, ...
      |     'bin_pt', bin_pt, ...
      |     'arith_type', arith_type);
      settings: AttributesFormatString=UFix_1_0
  Out1 : Outport
  Out2 : Outport  Port=2
  Out3 : Outport  Port=3
  Out4 : Outport  Port=4
  Out5 : Outport  Port=5
  Out6 : Outport  Port=6
  Out7 : Outport  Port=7
  Out8 : Outport  Port=8
  wires:
  Constant5/1 -> Mux/3
  Constant10/1 -> Mux1/3
  Mux/1 -> Out1/1
  Mux1/1 -> Out2/1
  Constant2/1 -> Mux2/3
  Constant1/1 -> Mux3/3
  Mux2/1 -> Out3/1
  Mux3/1 -> Out4/1
  In1/1 -> Mux/2
  In4/1 -> Mux3/2
  In2/1 -> Mux1/2
  In3/1 -> Mux2/2
  en_mask/1 -> uncram/1(In)
  Constant7/1 -> Mux4/3
  Constant4/1 -> Mux5/3
  Mux4/1 -> Out5/1
  Mux5/1 -> Out6/1
  Constant6/1 -> Mux6/3
  Constant3/1 -> Mux7/3
  Mux6/1 -> Out7/1
  Mux7/1 -> Out8/1
  In5/1 -> Mux4/2
  In6/1 -> Mux5/2
  In8/1 -> Mux7/2
  In7/1 -> Mux6/2
  uncram/1(Out1) -> Mux7/1
  uncram/2(Out2) -> Mux6/1
  uncram/3(Out3) -> Mux5/1
  uncram/4(Out4) -> Mux4/1
  uncram/5(Out5) -> Mux3/1
  uncram/6(Out6) -> Mux2/1
  uncram/7(Out7) -> Mux1/1
  uncram/8(Out8) -> Mux/1

== system x64_32s_001/16_power/convert_of ==
  din : Inport  OutDataType=sfix(16)  OutScaling=2^0
  convert : xbsIndex_r4/Convert  arith_type=Unsigned  n_bits=bit_width_o  bin_pt=binary_point_o  quantization="Round  (unbiased: +/- Inf)"  overflow=Saturate  latency=latency  dbl_ovrd=off  pipeline=on  block_type=convert
      settings: block_version=10.1.1
  never : xbsIndex_r4/Constant  arith_type=Boolean  const=0  n_bits=16  bin_pt=14  explicit_period=on  period=1  equ=P=C  opselect=C  inp2=PCIN>>17  opr=+  inp1=P  carry=CIN  dbl_ovrd=off  block_type=constant
      settings: block_version=11.4
  dout : Outport
  of : Outport  Port=2
  wires:
  din/1 -> convert/1
  convert/1 -> dout/1
  never/1 -> of/1
